simulink model "carcontroller"  (R2022b)
settings: Solver=FixedStepAuto  StartTime=0.0  StopTime=inf  RelTol=1e-3
model workspace (MAT data in the model file):
  Logfile_Data_Object : robotics.ros.BagSelection (value not decoded)
  Logfile_Path = '/Users/sprinkle/Library/CloudStorage/OneDrive-Vanderbilt/Presentations/DW_JS/TEACHING/Lecture Slides/2023_09_28_16_19_25_2T3MWRFVXLW056972microstrain_record.bag'
  Logfile_Playback_Duration = nan
  Logfile_Playback_Offset = 100
libraries: robotlib x3
settings Model: Creator=sprinkle  LastModifiedBy=sprinkle
settings SolverCC: AlgebraicLoopSolver=TrustRegion
settings OptimizationCC: EnhancedBackFolding=on  PassReuseOutputArgsAs=Individual arguments  ZeroExternalMemoryAtStartup=off  ZeroInternalMemoryAtStartup=off  MaxStackSize=64  OptimizeBlockOrder=speed  DifferentSizesBufferReuse=on  OptimizationCustomize=off  EfficientTunableParamExpr=on
settings DebuggingCC: ParameterTunabilityLossMsg=error  UnconnectedInputMsg=warning  UnconnectedOutputMsg=warning  UnconnectedLineMsg=warning  SFMachineParentedDataDiag=warning
settings HardwareCC: ProdBitPerPointer=32  ProdBitPerSizeT=32  ProdBitPerPtrDiffT=32  ProdLargestAtomicFloat=None  ProdIntDivRoundTo=Undefined  ProdEndianess=Unspecified  ProdWordSize=32  ProdHWDeviceType=Generic->Unspecified (assume 32-bit Generic)
settings ModelReferenceCC: EnableRefExpFcnMdlSchedulingChecks=on
settings SFSimCC: MATLABDynamicMemAlloc=off
settings RTWCC: Description=Embedded Coder  SystemTargetFile=ert.tlc  HardwareBoard=Robot Operating System (ROS)  TLCOptions="-aInlineSetEventsForThisBaseRateFcn=TLC_FALSE -aSuppressMultiTaskScheduler=TLC_FALSE  -aRateBasedStepFcn=0"  TemplateMakefile=ert_default_tmf  Toolchain=Catkin  TargetLang=C++
settings CodeAppCC: ForceParamTrailComments=on  IgnoreCustomStorageClasses=off  OperatorAnnotations=on  SimulinkDataObjDesc=on  SFDataObjDesc=on  InsertBlockDesc=on
settings ERTTargetCC: SupportContinuousTime=on

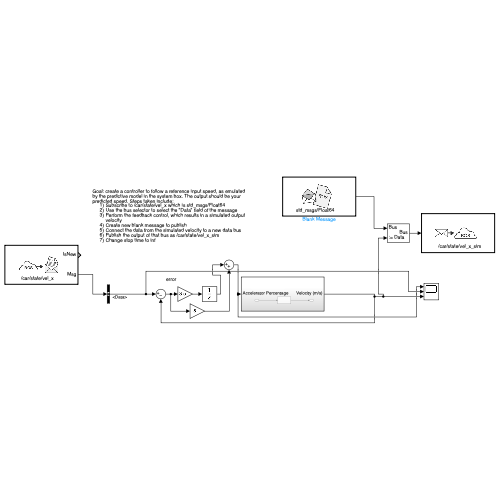
[thumbnail: Simulink's stored preview of the top-level diagram]
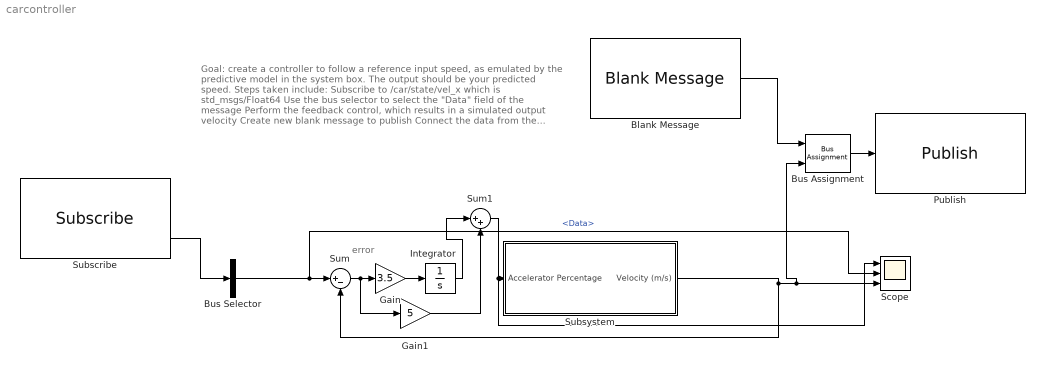
== system carcontroller ==
  note: error
  note: Goal: create a controller to follow a reference input speed, as emulated by the predictive model in the system box. The output should be your predicted speed. Steps taken include:
      | Subscribe to /car/state/vel_x which is std_msgs/Float64
      | Use the bus selector to select the "Data" field of the message
      | Perform the feedback control, which results in a simulated output velocity
      | Create new blank message to publish
      | Connect the data from the simulated velocity to a new data bus
      | Publish the output of that bus as /car/state/vel_x_sim
      | Change stop time to inf
  Blank Message : robotlib/Blank Message  messageClass=Message  entityType=std_msgs/Float64  messageType=std_msgs/Float64  SampleTime=inf  legacyBusStruct=off
      settings: LibrarySourceBlock=roslib/ROS/Blank Message
  Bus Assignment : BusAssignment  AssignedSignals=Data
  Bus Selector : BusSelector  OutputSignals=Data
  Gain : Gain  Gain=3.5
  Gain1 : Gain  Gain=5
  Integrator : Integrator
  Publish : robotlib/Publish  topicSource=Specify your own  topic=/car/state/vel_x_sim  messageType=std_msgs/Float64  legacyBusStruct=off  messageQueueLength=1
      settings: LibrarySourceBlock=roslib/ROS/Publish
  Scope : Scope  NumInputPorts=3
  Subscribe : robotlib/Subscribe  topicSource=Specify your own  topic=/car/state/vel_x  messageType=std_msgs/Float64  SampleTime=-1  legacyBusStruct=off  messageQueueLength=1
      settings: LibrarySourceBlock=roslib/ROS/Subscribe
  Subsystem : Subsystem
  Sum : Sum  Inputs=|+-
  Sum1 : Sum  Inputs=|++
  wires:
  Subsystem/1(Velocity (m/s)) -> Bus Assignment/2, Scope/3, Sum/2
  Bus Selector/1 -> Scope/2, Sum/1  "<Data>"
  Sum/1 -> Gain1/1, Gain/1
  Gain/1 -> Integrator/1
  Integrator/1 -> Sum1/1
  Sum1/1 -> Scope/1, Subsystem/1(Accelerator Percentage)
  Gain1/1 -> Sum1/2
  Subscribe/2 -> Bus Selector/1
  Bus Assignment/1 -> Publish/1
  Blank Message/1 -> Bus Assignment/1

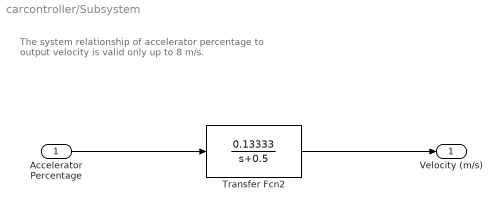
== system carcontroller/Subsystem ==
  note: The system relationship of accelerator percentage to output velocity is valid only up to 8 m/s.
  Accelerator Percentage : Inport
  Transfer Fcn2 : TransferFcn  Numerator=[0.13333]  Denominator=[1 0.5]
  Velocity (m/s) : Outport
  wires:
  Accelerator Percentage/1 -> Transfer Fcn2/1
  Transfer Fcn2/1 -> Velocity (m/s)/1
